simulink model "wind1000_BUS_Original"  (R2024a) (continued)
== system wind1000_BUS_Original/Subsystem_10/String1/WT6 ==
  V_in : Inport
  I_in : Inport  Port=2
  Add1 : Sum  Inputs=+-
  C_cable : Subsystem
  C_filter : Subsystem
  Cable line : Subsystem
  Gain : Gain  Gain=1/parGrid.WTstring.Rparallel_cable
  Transformer-complex : Subsystem
  WFG : Subsystem
  V_out : Outport
  I_out : Outport  Port=2
  wires:
  I_in/1 -> C_cable/2(I_in)
  Gain/1 -> Add1/2
  Add1/1 -> C_cable/1(It2)
  Transformer-complex/2(It2) -> Add1/1
  V_in/1 -> Cable line/1(V)
  Transformer-complex/1(It1) -> C_filter/2(It1)
  Cable line/1(I_cable) -> C_cable/3(I_cable), I_out/1
  C_filter/1(Vc_filter_qd) -> WFG/1(vcqd), Transformer-complex/1(Vc_filter_qd)
  WFG/1(ilqd) -> C_filter/1(IWF)
  C_cable/1(Vc_cable) -> Cable line/2(Vc_cable), V_out/1, Transformer-complex/2(Vc_cable_qd), Gain/1

== system wind1000_BUS_Original/Subsystem_10/String1/WT7 ==
  V_in : Inport
  I_in : Inport  Port=2
  Add1 : Sum  Inputs=+-
  C_cable : Subsystem
  C_filter : Subsystem
  Cable line : Subsystem
  Gain : Gain  Gain=1/parGrid.WTstring.Rparallel_cable
  Transformer-complex : Subsystem
  WFG : Subsystem
  V_out : Outport
  I_out : Outport  Port=2
  wires:
  C_cable/1(Vc_cable) -> Gain/1, Transformer-complex/2(Vc_cable_qd), V_out/1, Cable line/2(Vc_cable)
  WFG/1(ilqd) -> C_filter/1(IWF)
  C_filter/1(Vc_filter_qd) -> WFG/1(vcqd), Transformer-complex/1(Vc_filter_qd)
  Cable line/1(I_cable) -> I_out/1, C_cable/3(I_cable)
  Transformer-complex/1(It1) -> C_filter/2(It1)
  V_in/1 -> Cable line/1(V)
  Transformer-complex/2(It2) -> Add1/1
  Add1/1 -> C_cable/1(It2)
  Gain/1 -> Add1/2
  I_in/1 -> C_cable/2(I_in)

== system wind1000_BUS_Original/Subsystem_10/String1/WT8 ==
  V_in : Inport
  I_in : Inport  Port=2
  Add1 : Sum  Inputs=+-
  C_cable : Subsystem
  C_filter : Subsystem
  Cable line : Subsystem
  Gain : Gain  Gain=1/parGrid.WTstring.Rparallel_cable
  Transformer-complex : Subsystem
  WFG : Subsystem
  V_out : Outport
  I_out : Outport  Port=2
  wires:
  I_in/1 -> C_cable/2(I_in)
  Gain/1 -> Add1/2
  Add1/1 -> C_cable/1(It2)
  Transformer-complex/2(It2) -> Add1/1
  V_in/1 -> Cable line/1(V)
  Transformer-complex/1(It1) -> C_filter/2(It1)
  Cable line/1(I_cable) -> C_cable/3(I_cable), I_out/1
  C_filter/1(Vc_filter_qd) -> WFG/1(vcqd), Transformer-complex/1(Vc_filter_qd)
  WFG/1(ilqd) -> C_filter/1(IWF)
  C_cable/1(Vc_cable) -> Cable line/2(Vc_cable), V_out/1, Transformer-complex/2(Vc_cable_qd), Gain/1

== system wind1000_BUS_Original/Subsystem_10/String1/WT9 ==
  V_in : Inport
  I_in : Inport  Port=2
  Add1 : Sum  Inputs=+-
  C_cable : Subsystem
  C_filter : Subsystem
  Cable line : Subsystem
  Gain : Gain  Gain=1/parGrid.WTstring.Rparallel_cable
  Transformer-complex : Subsystem
  WFG : Subsystem
  V_out : Outport
  I_out : Outport  Port=2
  wires:
  C_cable/1(Vc_cable) -> Gain/1, Transformer-complex/2(Vc_cable_qd), V_out/1, Cable line/2(Vc_cable)
  WFG/1(ilqd) -> C_filter/1(IWF)
  C_filter/1(Vc_filter_qd) -> WFG/1(vcqd), Transformer-complex/1(Vc_filter_qd)
  Cable line/1(I_cable) -> I_out/1, C_cable/3(I_cable)
  Transformer-complex/1(It1) -> C_filter/2(It1)
  V_in/1 -> Cable line/1(V)
  Transformer-complex/2(It2) -> Add1/1
  Add1/1 -> C_cable/1(It2)
  Gain/1 -> Add1/2
  I_in/1 -> C_cable/2(I_in)

== system wind1000_BUS_Original/Subsystem_20/3phase2dq/Font trifasica asimetrica1 ==
  t : Inport
  Clock : Clock
  Constant1 : Constant  Value=phi_D
  Constant2 : Constant  Value=phi_I
  Constant3 : Constant  Value=2*pi/3
  Constant4 : Constant  Value=2*pi/3
  Gain : Gain  Gain=we
  Gain1 : Gain  Gain=A_D
  Gain2 : Gain  Gain=A_I
  Mux : Mux  Inputs=3
  Mux1 : Mux  Inputs=3
  Park : Subsystem
  Product : Product  RndMeth=Zero
  Product1 : Product  RndMeth=Zero
  Product4 : Product  Multiplication=Matrix(*)  RndMeth=Zero
  Scope1 : Scope
  Sum{1,3,5..7} (5 alike) : Sum  Inputs=|++
  Sum2 : Sum  Inputs=|+-
  Sum4 : Sum  Inputs=|+-
  Trigonometric Function{1..6} (6 alike) : Trigonometry  Operator=cos
  directa : Lookup  InputValues=tt_D  Table=tA_D  SaturateOnIntegerOverflow=off
  inversa : Lookup  InputValues=tt_I  Table=tA_I  SaturateOnIntegerOverflow=off
  we : Gain  Gain=100*pi
  y : Outport
  wires:
  Sum3/1 -> Trigonometric Function2/1, Sum2/1, Sum1/1
  Sum2/1 -> Trigonometric Function1/1
  Sum1/1 -> Trigonometric Function3/1
  Constant4/1 -> Sum2/2, Sum1/2
  Trigonometric Function2/1 -> Mux/1
  Trigonometric Function1/1 -> Mux/2
  Trigonometric Function3/1 -> Mux/3
  Mux/1 -> Gain1/1
  t/1 -> Gain/1, directa/1, inversa/1
  Gain/1 -> Sum3/1, Sum6/1
  Constant1/1 -> Sum3/2
  Sum6/1 -> Trigonometric Function5/1, Sum5/1, Sum4/1
  Sum5/1 -> Trigonometric Function4/1
  Sum4/1 -> Trigonometric Function6/1
  Constant3/1 -> Sum4/2, Sum5/2
  Trigonometric Function5/1 -> Mux1/1
  Trigonometric Function4/1 -> Mux1/2
  Trigonometric Function6/1 -> Mux1/3
  Mux1/1 -> Gain2/1
  Constant2/1 -> Sum6/2
  Product/1 -> Sum7/1
  directa/1 -> Product/1
  inversa/1 -> Product1/1
  Gain1/1 -> Product/2
  Gain2/1 -> Product1/2
  Product1/1 -> Sum7/2
  Park/1(Tthetar) -> Scope1/1, Product4/1
  Clock/1 -> we/1
  we/1 -> Park/1(theta_r)
  Sum7/1 -> Product4/2
  Product4/1 -> y/1

== system wind1000_BUS_Original/Subsystem_20/Subsystem/String1 ==
  V_in : Inport
  Constant : Constant  Value=0
  Scope : Scope
  WT1 : Subsystem
  WT10 : Subsystem
  WT2 : Subsystem
  WT3 : Subsystem
  WT4 : Subsystem
  WT5 : Subsystem
  WT6 : Subsystem
  WT7 : Subsystem
  WT8 : Subsystem
  WT9 : Subsystem
  I_out : Outport
  wires:
  WT10/1(V_out) -> Scope/1
  WT7/1(V_out) -> WT8/1(V_in)
  Constant/1 -> WT10/2(I_in)
  WT4/1(V_out) -> WT5/1(V_in)
  WT4/2(I_out) -> WT3/2(I_in)
  WT3/1(V_out) -> WT4/1(V_in)
  WT1/1(V_out) -> WT2/1(V_in)
  WT10/2(I_out) -> WT9/2(I_in)
  WT5/1(V_out) -> WT6/1(V_in)
  WT2/1(V_out) -> WT3/1(V_in)
  WT7/2(I_out) -> WT6/2(I_in)
  WT8/1(V_out) -> WT9/1(V_in)
  WT6/1(V_out) -> WT7/1(V_in)
  WT9/2(I_out) -> WT8/2(I_in)
  WT5/2(I_out) -> WT4/2(I_in)
  WT2/2(I_out) -> WT1/2(I_in)
  V_in/1 -> WT1/1(V_in)
  WT3/2(I_out) -> WT2/2(I_in)
  WT8/2(I_out) -> WT7/2(I_in)
  WT9/1(V_out) -> WT10/1(V_in)
  WT6/2(I_out) -> WT5/2(I_in)
  WT1/2(I_out) -> I_out/1

== system wind1000_BUS_Original/Subsystem_20/Subsystem/String2 ==
  V_in : Inport
  Constant : Constant  Value=0
  Scope : Scope
  WT1 : Subsystem
  WT10 : Subsystem
  WT2 : Subsystem
  WT3 : Subsystem
  WT4 : Subsystem
  WT5 : Subsystem
  WT6 : Subsystem
  WT7 : Subsystem
  WT8 : Subsystem
  WT9 : Subsystem
  I_out : Outport
  wires:
  WT1/2(I_out) -> I_out/1
  WT6/2(I_out) -> WT5/2(I_in)
  WT9/1(V_out) -> WT10/1(V_in)
  WT8/2(I_out) -> WT7/2(I_in)
  WT3/2(I_out) -> WT2/2(I_in)
  V_in/1 -> WT1/1(V_in)
  WT2/2(I_out) -> WT1/2(I_in)
  WT5/2(I_out) -> WT4/2(I_in)
  WT9/2(I_out) -> WT8/2(I_in)
  WT6/1(V_out) -> WT7/1(V_in)
  WT8/1(V_out) -> WT9/1(V_in)
  WT7/2(I_out) -> WT6/2(I_in)
  WT2/1(V_out) -> WT3/1(V_in)
  WT5/1(V_out) -> WT6/1(V_in)
  WT10/2(I_out) -> WT9/2(I_in)
  WT1/1(V_out) -> WT2/1(V_in)
  WT3/1(V_out) -> WT4/1(V_in)
  WT4/2(I_out) -> WT3/2(I_in)
  WT4/1(V_out) -> WT5/1(V_in)
  Constant/1 -> WT10/2(I_in)
  WT7/1(V_out) -> WT8/1(V_in)
  WT10/1(V_out) -> Scope/1

== system wind1000_BUS_Original/Subsystem_20/Subsystem/String3 ==
  V_in : Inport
  Constant : Constant  Value=0
  Scope : Scope
  WT1 : Subsystem
  WT10 : Subsystem
  WT2 : Subsystem
  WT3 : Subsystem
  WT4 : Subsystem
  WT5 : Subsystem
  WT6 : Subsystem
  WT7 : Subsystem
  WT8 : Subsystem
  WT9 : Subsystem
  I_out : Outport
  wires:
  WT1/2(I_out) -> I_out/1
  WT6/2(I_out) -> WT5/2(I_in)
  WT9/1(V_out) -> WT10/1(V_in)
  WT8/2(I_out) -> WT7/2(I_in)
  WT3/2(I_out) -> WT2/2(I_in)
  V_in/1 -> WT1/1(V_in)
  WT2/2(I_out) -> WT1/2(I_in)
  WT5/2(I_out) -> WT4/2(I_in)
  WT9/2(I_out) -> WT8/2(I_in)
  WT6/1(V_out) -> WT7/1(V_in)
  WT8/1(V_out) -> WT9/1(V_in)
  WT7/2(I_out) -> WT6/2(I_in)
  WT2/1(V_out) -> WT3/1(V_in)
  WT5/1(V_out) -> WT6/1(V_in)
  WT10/2(I_out) -> WT9/2(I_in)
  WT1/1(V_out) -> WT2/1(V_in)
  WT3/1(V_out) -> WT4/1(V_in)
  WT4/2(I_out) -> WT3/2(I_in)
  WT4/1(V_out) -> WT5/1(V_in)
  Constant/1 -> WT10/2(I_in)
  WT7/1(V_out) -> WT8/1(V_in)
  WT10/1(V_out) -> Scope/1

== system wind1000_BUS_Original/Subsystem_20/Subsystem/String4 ==
  V_in : Inport
  Constant : Constant  Value=0
  Scope : Scope
  WT1 : Subsystem
  WT10 : Subsystem
  WT2 : Subsystem
  WT3 : Subsystem
  WT4 : Subsystem
  WT5 : Subsystem
  WT6 : Subsystem
  WT7 : Subsystem
  WT8 : Subsystem
  WT9 : Subsystem
  I_out : Outport
  wires:
  WT1/2(I_out) -> I_out/1
  WT6/2(I_out) -> WT5/2(I_in)
  WT9/1(V_out) -> WT10/1(V_in)
  WT8/2(I_out) -> WT7/2(I_in)
  WT3/2(I_out) -> WT2/2(I_in)
  V_in/1 -> WT1/1(V_in)
  WT2/2(I_out) -> WT1/2(I_in)
  WT5/2(I_out) -> WT4/2(I_in)
  WT9/2(I_out) -> WT8/2(I_in)
  WT6/1(V_out) -> WT7/1(V_in)
  WT8/1(V_out) -> WT9/1(V_in)
  WT7/2(I_out) -> WT6/2(I_in)
  WT2/1(V_out) -> WT3/1(V_in)
  WT5/1(V_out) -> WT6/1(V_in)
  WT10/2(I_out) -> WT9/2(I_in)
  WT1/1(V_out) -> WT2/1(V_in)
  WT3/1(V_out) -> WT4/1(V_in)
  WT4/2(I_out) -> WT3/2(I_in)
  WT4/1(V_out) -> WT5/1(V_in)
  Constant/1 -> WT10/2(I_in)
  WT7/1(V_out) -> WT8/1(V_in)
  WT10/1(V_out) -> Scope/1

== system wind1000_BUS_Original/Subsystem_20/Subsystem/String5 ==
  V_in : Inport
  Constant : Constant  Value=0
  Scope : Scope
  WT1 : Subsystem
  WT10 : Subsystem
  WT2 : Subsystem
  WT3 : Subsystem
  WT4 : Subsystem
  WT5 : Subsystem
  WT6 : Subsystem
  WT7 : Subsystem
  WT8 : Subsystem
  WT9 : Subsystem
  I_out : Outport
  wires:
  WT1/2(I_out) -> I_out/1
  WT6/2(I_out) -> WT5/2(I_in)
  WT9/1(V_out) -> WT10/1(V_in)
  WT8/2(I_out) -> WT7/2(I_in)
  WT3/2(I_out) -> WT2/2(I_in)
  V_in/1 -> WT1/1(V_in)
  WT2/2(I_out) -> WT1/2(I_in)
  WT5/2(I_out) -> WT4/2(I_in)
  WT9/2(I_out) -> WT8/2(I_in)
  WT6/1(V_out) -> WT7/1(V_in)
  WT8/1(V_out) -> WT9/1(V_in)
  WT7/2(I_out) -> WT6/2(I_in)
  WT2/1(V_out) -> WT3/1(V_in)
  WT5/1(V_out) -> WT6/1(V_in)
  WT10/2(I_out) -> WT9/2(I_in)
  WT1/1(V_out) -> WT2/1(V_in)
  WT3/1(V_out) -> WT4/1(V_in)
  WT4/2(I_out) -> WT3/2(I_in)
  WT4/1(V_out) -> WT5/1(V_in)
  Constant/1 -> WT10/2(I_in)
  WT7/1(V_out) -> WT8/1(V_in)
  WT10/1(V_out) -> Scope/1

== system wind1000_BUS_Original/Subsystem_20/Subsystem/String6 ==
  V_in : Inport
  Constant : Constant  Value=0
  Scope : Scope
  WT1 : Subsystem
  WT10 : Subsystem
  WT2 : Subsystem
  WT3 : Subsystem
  WT4 : Subsystem
  WT5 : Subsystem
  WT6 : Subsystem
  WT7 : Subsystem
  WT8 : Subsystem
  WT9 : Subsystem
  I_out : Outport
  wires:
  WT1/2(I_out) -> I_out/1
  WT6/2(I_out) -> WT5/2(I_in)
  WT9/1(V_out) -> WT10/1(V_in)
  WT8/2(I_out) -> WT7/2(I_in)
  WT3/2(I_out) -> WT2/2(I_in)
  V_in/1 -> WT1/1(V_in)
  WT2/2(I_out) -> WT1/2(I_in)
  WT5/2(I_out) -> WT4/2(I_in)
  WT9/2(I_out) -> WT8/2(I_in)
  WT6/1(V_out) -> WT7/1(V_in)
  WT8/1(V_out) -> WT9/1(V_in)
  WT7/2(I_out) -> WT6/2(I_in)
  WT2/1(V_out) -> WT3/1(V_in)
  WT5/1(V_out) -> WT6/1(V_in)
  WT10/2(I_out) -> WT9/2(I_in)
  WT1/1(V_out) -> WT2/1(V_in)
  WT3/1(V_out) -> WT4/1(V_in)
  WT4/2(I_out) -> WT3/2(I_in)
  WT4/1(V_out) -> WT5/1(V_in)
  Constant/1 -> WT10/2(I_in)
  WT7/1(V_out) -> WT8/1(V_in)
  WT10/1(V_out) -> Scope/1

== system wind1000_BUS_Original/Subsystem_200/3phase2dq/Font trifasica asimetrica1 ==
  t : Inport
  Clock : Clock
  Constant1 : Constant  Value=phi_D
  Constant2 : Constant  Value=phi_I
  Constant3 : Constant  Value=2*pi/3
  Constant4 : Constant  Value=2*pi/3
  Gain : Gain  Gain=we
  Gain1 : Gain  Gain=A_D
  Gain2 : Gain  Gain=A_I
  Mux : Mux  Inputs=3
  Mux1 : Mux  Inputs=3
  Park : Subsystem
  Product : Product  RndMeth=Zero
  Product1 : Product  RndMeth=Zero
  Product4 : Product  Multiplication=Matrix(*)  RndMeth=Zero
  Scope1 : Scope
  Sum{1,3,5..7} (5 alike) : Sum  Inputs=|++
  Sum2 : Sum  Inputs=|+-
  Sum4 : Sum  Inputs=|+-
  Trigonometric Function{1..6} (6 alike) : Trigonometry  Operator=cos
  directa : Lookup  InputValues=tt_D  Table=tA_D  SaturateOnIntegerOverflow=off
  inversa : Lookup  InputValues=tt_I  Table=tA_I  SaturateOnIntegerOverflow=off
  we : Gain  Gain=100*pi
  y : Outport
  wires:
  Sum3/1 -> Trigonometric Function2/1, Sum2/1, Sum1/1
  Sum2/1 -> Trigonometric Function1/1
  Sum1/1 -> Trigonometric Function3/1
  Constant4/1 -> Sum2/2, Sum1/2
  Trigonometric Function2/1 -> Mux/1
  Trigonometric Function1/1 -> Mux/2
  Trigonometric Function3/1 -> Mux/3
  Mux/1 -> Gain1/1
  t/1 -> Gain/1, directa/1, inversa/1
  Gain/1 -> Sum3/1, Sum6/1
  Constant1/1 -> Sum3/2
  Sum6/1 -> Trigonometric Function5/1, Sum5/1, Sum4/1
  Sum5/1 -> Trigonometric Function4/1
  Sum4/1 -> Trigonometric Function6/1
  Constant3/1 -> Sum4/2, Sum5/2
  Trigonometric Function5/1 -> Mux1/1
  Trigonometric Function4/1 -> Mux1/2
  Trigonometric Function6/1 -> Mux1/3
  Mux1/1 -> Gain2/1
  Constant2/1 -> Sum6/2
  Product/1 -> Sum7/1
  directa/1 -> Product/1
  inversa/1 -> Product1/1
  Gain1/1 -> Product/2
  Gain2/1 -> Product1/2
  Product1/1 -> Sum7/2
  Park/1(Tthetar) -> Scope1/1, Product4/1
  Clock/1 -> we/1
  we/1 -> Park/1(theta_r)
  Sum7/1 -> Product4/2
  Product4/1 -> y/1

== system wind1000_BUS_Original/Subsystem_200/Subsystem/String1 ==
  V_in : Inport
  Constant : Constant  Value=0
  Scope : Scope
  WT1 : Subsystem
  WT10 : Subsystem
  WT2 : Subsystem
  WT3 : Subsystem
  WT4 : Subsystem
  WT5 : Subsystem
  WT6 : Subsystem
  WT7 : Subsystem
  WT8 : Subsystem
  WT9 : Subsystem
  I_out : Outport
  wires:
  WT10/1(V_out) -> Scope/1
  WT7/1(V_out) -> WT8/1(V_in)
  Constant/1 -> WT10/2(I_in)
  WT4/1(V_out) -> WT5/1(V_in)
  WT4/2(I_out) -> WT3/2(I_in)
  WT3/1(V_out) -> WT4/1(V_in)
  WT1/1(V_out) -> WT2/1(V_in)
  WT10/2(I_out) -> WT9/2(I_in)
  WT5/1(V_out) -> WT6/1(V_in)
  WT2/1(V_out) -> WT3/1(V_in)
  WT7/2(I_out) -> WT6/2(I_in)
  WT8/1(V_out) -> WT9/1(V_in)
  WT6/1(V_out) -> WT7/1(V_in)
  WT9/2(I_out) -> WT8/2(I_in)
  WT5/2(I_out) -> WT4/2(I_in)
  WT2/2(I_out) -> WT1/2(I_in)
  V_in/1 -> WT1/1(V_in)
  WT3/2(I_out) -> WT2/2(I_in)
  WT8/2(I_out) -> WT7/2(I_in)
  WT9/1(V_out) -> WT10/1(V_in)
  WT6/2(I_out) -> WT5/2(I_in)
  WT1/2(I_out) -> I_out/1

== system wind1000_BUS_Original/Subsystem_200/Subsystem/String10 ==
  V_in : Inport
  Constant : Constant  Value=0
  Scope : Scope
  WT1 : Subsystem
  WT10 : Subsystem
  WT2 : Subsystem
  WT3 : Subsystem
  WT4 : Subsystem
  WT5 : Subsystem
  WT6 : Subsystem
  WT7 : Subsystem
  WT8 : Subsystem
  WT9 : Subsystem
  I_out : Outport
  wires:
  WT1/2(I_out) -> I_out/1
  WT6/2(I_out) -> WT5/2(I_in)
  WT9/1(V_out) -> WT10/1(V_in)
  WT8/2(I_out) -> WT7/2(I_in)
  WT3/2(I_out) -> WT2/2(I_in)
  V_in/1 -> WT1/1(V_in)
  WT2/2(I_out) -> WT1/2(I_in)
  WT5/2(I_out) -> WT4/2(I_in)
  WT9/2(I_out) -> WT8/2(I_in)
  WT6/1(V_out) -> WT7/1(V_in)
  WT8/1(V_out) -> WT9/1(V_in)
  WT7/2(I_out) -> WT6/2(I_in)
  WT2/1(V_out) -> WT3/1(V_in)
  WT5/1(V_out) -> WT6/1(V_in)
  WT10/2(I_out) -> WT9/2(I_in)
  WT1/1(V_out) -> WT2/1(V_in)
  WT3/1(V_out) -> WT4/1(V_in)
  WT4/2(I_out) -> WT3/2(I_in)
  WT4/1(V_out) -> WT5/1(V_in)
  Constant/1 -> WT10/2(I_in)
  WT7/1(V_out) -> WT8/1(V_in)
  WT10/1(V_out) -> Scope/1

== system wind1000_BUS_Original/Subsystem_200/Subsystem/String11 ==
  V_in : Inport
  Constant : Constant  Value=0
  Scope : Scope
  WT1 : Subsystem
  WT10 : Subsystem
  WT2 : Subsystem
  WT3 : Subsystem
  WT4 : Subsystem
  WT5 : Subsystem
  WT6 : Subsystem
  WT7 : Subsystem
  WT8 : Subsystem
  WT9 : Subsystem
  I_out : Outport
  wires:
  WT1/2(I_out) -> I_out/1
  WT6/2(I_out) -> WT5/2(I_in)
  WT9/1(V_out) -> WT10/1(V_in)
  WT8/2(I_out) -> WT7/2(I_in)
  WT3/2(I_out) -> WT2/2(I_in)
  V_in/1 -> WT1/1(V_in)
  WT2/2(I_out) -> WT1/2(I_in)
  WT5/2(I_out) -> WT4/2(I_in)
  WT9/2(I_out) -> WT8/2(I_in)
  WT6/1(V_out) -> WT7/1(V_in)
  WT8/1(V_out) -> WT9/1(V_in)
  WT7/2(I_out) -> WT6/2(I_in)
  WT2/1(V_out) -> WT3/1(V_in)
  WT5/1(V_out) -> WT6/1(V_in)
  WT10/2(I_out) -> WT9/2(I_in)
  WT1/1(V_out) -> WT2/1(V_in)
  WT3/1(V_out) -> WT4/1(V_in)
  WT4/2(I_out) -> WT3/2(I_in)
  WT4/1(V_out) -> WT5/1(V_in)
  Constant/1 -> WT10/2(I_in)
  WT7/1(V_out) -> WT8/1(V_in)
  WT10/1(V_out) -> Scope/1

== system wind1000_BUS_Original/Subsystem_200/Subsystem/String12 ==
  V_in : Inport
  Constant : Constant  Value=0
  Scope : Scope
  WT1 : Subsystem
  WT10 : Subsystem
  WT2 : Subsystem
  WT3 : Subsystem
  WT4 : Subsystem
  WT5 : Subsystem
  WT6 : Subsystem
  WT7 : Subsystem
  WT8 : Subsystem
  WT9 : Subsystem
  I_out : Outport
  wires:
  WT1/2(I_out) -> I_out/1
  WT6/2(I_out) -> WT5/2(I_in)
  WT9/1(V_out) -> WT10/1(V_in)
  WT8/2(I_out) -> WT7/2(I_in)
  WT3/2(I_out) -> WT2/2(I_in)
  V_in/1 -> WT1/1(V_in)
  WT2/2(I_out) -> WT1/2(I_in)
  WT5/2(I_out) -> WT4/2(I_in)
  WT9/2(I_out) -> WT8/2(I_in)
  WT6/1(V_out) -> WT7/1(V_in)
  WT8/1(V_out) -> WT9/1(V_in)
  WT7/2(I_out) -> WT6/2(I_in)
  WT2/1(V_out) -> WT3/1(V_in)
  WT5/1(V_out) -> WT6/1(V_in)
  WT10/2(I_out) -> WT9/2(I_in)
  WT1/1(V_out) -> WT2/1(V_in)
  WT3/1(V_out) -> WT4/1(V_in)
  WT4/2(I_out) -> WT3/2(I_in)
  WT4/1(V_out) -> WT5/1(V_in)
  Constant/1 -> WT10/2(I_in)
  WT7/1(V_out) -> WT8/1(V_in)
  WT10/1(V_out) -> Scope/1

== system wind1000_BUS_Original/Subsystem_200/Subsystem/String13 ==
  V_in : Inport
  Constant : Constant  Value=0
  Scope : Scope
  WT1 : Subsystem
  WT10 : Subsystem
  WT2 : Subsystem
  WT3 : Subsystem
  WT4 : Subsystem
  WT5 : Subsystem
  WT6 : Subsystem
  WT7 : Subsystem
  WT8 : Subsystem
  WT9 : Subsystem
  I_out : Outport
  wires:
  WT1/2(I_out) -> I_out/1
  WT6/2(I_out) -> WT5/2(I_in)
  WT9/1(V_out) -> WT10/1(V_in)
  WT8/2(I_out) -> WT7/2(I_in)
  WT3/2(I_out) -> WT2/2(I_in)
  V_in/1 -> WT1/1(V_in)
  WT2/2(I_out) -> WT1/2(I_in)
  WT5/2(I_out) -> WT4/2(I_in)
  WT9/2(I_out) -> WT8/2(I_in)
  WT6/1(V_out) -> WT7/1(V_in)
  WT8/1(V_out) -> WT9/1(V_in)
  WT7/2(I_out) -> WT6/2(I_in)
  WT2/1(V_out) -> WT3/1(V_in)
  WT5/1(V_out) -> WT6/1(V_in)
  WT10/2(I_out) -> WT9/2(I_in)
  WT1/1(V_out) -> WT2/1(V_in)
  WT3/1(V_out) -> WT4/1(V_in)
  WT4/2(I_out) -> WT3/2(I_in)
  WT4/1(V_out) -> WT5/1(V_in)
  Constant/1 -> WT10/2(I_in)
  WT7/1(V_out) -> WT8/1(V_in)
  WT10/1(V_out) -> Scope/1

== system wind1000_BUS_Original/Subsystem_200/Subsystem/String14 ==
  V_in : Inport
  Constant : Constant  Value=0
  Scope : Scope
  WT1 : Subsystem
  WT10 : Subsystem
  WT2 : Subsystem
  WT3 : Subsystem
  WT4 : Subsystem
  WT5 : Subsystem
  WT6 : Subsystem
  WT7 : Subsystem
  WT8 : Subsystem
  WT9 : Subsystem
  I_out : Outport
  wires:
  WT1/2(I_out) -> I_out/1
  WT6/2(I_out) -> WT5/2(I_in)
  WT9/1(V_out) -> WT10/1(V_in)
  WT8/2(I_out) -> WT7/2(I_in)
  WT3/2(I_out) -> WT2/2(I_in)
  V_in/1 -> WT1/1(V_in)
  WT2/2(I_out) -> WT1/2(I_in)
  WT5/2(I_out) -> WT4/2(I_in)
  WT9/2(I_out) -> WT8/2(I_in)
  WT6/1(V_out) -> WT7/1(V_in)
  WT8/1(V_out) -> WT9/1(V_in)
  WT7/2(I_out) -> WT6/2(I_in)
  WT2/1(V_out) -> WT3/1(V_in)
  WT5/1(V_out) -> WT6/1(V_in)
  WT10/2(I_out) -> WT9/2(I_in)
  WT1/1(V_out) -> WT2/1(V_in)
  WT3/1(V_out) -> WT4/1(V_in)
  WT4/2(I_out) -> WT3/2(I_in)
  WT4/1(V_out) -> WT5/1(V_in)
  Constant/1 -> WT10/2(I_in)
  WT7/1(V_out) -> WT8/1(V_in)
  WT10/1(V_out) -> Scope/1

== system wind1000_BUS_Original/Subsystem_200/Subsystem/String15 ==
  V_in : Inport
  Constant : Constant  Value=0
  Scope : Scope
  WT1 : Subsystem
  WT10 : Subsystem
  WT2 : Subsystem
  WT3 : Subsystem
  WT4 : Subsystem
  WT5 : Subsystem
  WT6 : Subsystem
  WT7 : Subsystem
  WT8 : Subsystem
  WT9 : Subsystem
  I_out : Outport
  wires:
  WT1/2(I_out) -> I_out/1
  WT6/2(I_out) -> WT5/2(I_in)
  WT9/1(V_out) -> WT10/1(V_in)
  WT8/2(I_out) -> WT7/2(I_in)
  WT3/2(I_out) -> WT2/2(I_in)
  V_in/1 -> WT1/1(V_in)
  WT2/2(I_out) -> WT1/2(I_in)
  WT5/2(I_out) -> WT4/2(I_in)
  WT9/2(I_out) -> WT8/2(I_in)
  WT6/1(V_out) -> WT7/1(V_in)
  WT8/1(V_out) -> WT9/1(V_in)
  WT7/2(I_out) -> WT6/2(I_in)
  WT2/1(V_out) -> WT3/1(V_in)
  WT5/1(V_out) -> WT6/1(V_in)
  WT10/2(I_out) -> WT9/2(I_in)
  WT1/1(V_out) -> WT2/1(V_in)
  WT3/1(V_out) -> WT4/1(V_in)
  WT4/2(I_out) -> WT3/2(I_in)
  WT4/1(V_out) -> WT5/1(V_in)
  Constant/1 -> WT10/2(I_in)
  WT7/1(V_out) -> WT8/1(V_in)
  WT10/1(V_out) -> Scope/1

== system wind1000_BUS_Original/Subsystem_200/Subsystem/String16 ==
  V_in : Inport
  Constant : Constant  Value=0
  Scope : Scope
  WT1 : Subsystem
  WT10 : Subsystem
  WT2 : Subsystem
  WT3 : Subsystem
  WT4 : Subsystem
  WT5 : Subsystem
  WT6 : Subsystem
  WT7 : Subsystem
  WT8 : Subsystem
  WT9 : Subsystem
  I_out : Outport
  wires:
  WT1/2(I_out) -> I_out/1
  WT6/2(I_out) -> WT5/2(I_in)
  WT9/1(V_out) -> WT10/1(V_in)
  WT8/2(I_out) -> WT7/2(I_in)
  WT3/2(I_out) -> WT2/2(I_in)
  V_in/1 -> WT1/1(V_in)
  WT2/2(I_out) -> WT1/2(I_in)
  WT5/2(I_out) -> WT4/2(I_in)
  WT9/2(I_out) -> WT8/2(I_in)
  WT6/1(V_out) -> WT7/1(V_in)
  WT8/1(V_out) -> WT9/1(V_in)
  WT7/2(I_out) -> WT6/2(I_in)
  WT2/1(V_out) -> WT3/1(V_in)
  WT5/1(V_out) -> WT6/1(V_in)
  WT10/2(I_out) -> WT9/2(I_in)
  WT1/1(V_out) -> WT2/1(V_in)
  WT3/1(V_out) -> WT4/1(V_in)
  WT4/2(I_out) -> WT3/2(I_in)
  WT4/1(V_out) -> WT5/1(V_in)
  Constant/1 -> WT10/2(I_in)
  WT7/1(V_out) -> WT8/1(V_in)
  WT10/1(V_out) -> Scope/1

== system wind1000_BUS_Original/Subsystem_200/Subsystem/String17 ==
  V_in : Inport
  Constant : Constant  Value=0
  Scope : Scope
  WT1 : Subsystem
  WT10 : Subsystem
  WT2 : Subsystem
  WT3 : Subsystem
  WT4 : Subsystem
  WT5 : Subsystem
  WT6 : Subsystem
  WT7 : Subsystem
  WT8 : Subsystem
  WT9 : Subsystem
  I_out : Outport
  wires:
  WT1/2(I_out) -> I_out/1
  WT6/2(I_out) -> WT5/2(I_in)
  WT9/1(V_out) -> WT10/1(V_in)
  WT8/2(I_out) -> WT7/2(I_in)
  WT3/2(I_out) -> WT2/2(I_in)
  V_in/1 -> WT1/1(V_in)
  WT2/2(I_out) -> WT1/2(I_in)
  WT5/2(I_out) -> WT4/2(I_in)
  WT9/2(I_out) -> WT8/2(I_in)
  WT6/1(V_out) -> WT7/1(V_in)
  WT8/1(V_out) -> WT9/1(V_in)
  WT7/2(I_out) -> WT6/2(I_in)
  WT2/1(V_out) -> WT3/1(V_in)
  WT5/1(V_out) -> WT6/1(V_in)
  WT10/2(I_out) -> WT9/2(I_in)
  WT1/1(V_out) -> WT2/1(V_in)
  WT3/1(V_out) -> WT4/1(V_in)
  WT4/2(I_out) -> WT3/2(I_in)
  WT4/1(V_out) -> WT5/1(V_in)
  Constant/1 -> WT10/2(I_in)
  WT7/1(V_out) -> WT8/1(V_in)
  WT10/1(V_out) -> Scope/1

== system wind1000_BUS_Original/Subsystem_200/Subsystem/String18 ==
  V_in : Inport
  Constant : Constant  Value=0
  Scope : Scope
  WT1 : Subsystem
  WT10 : Subsystem
  WT2 : Subsystem
  WT3 : Subsystem
  WT4 : Subsystem
  WT5 : Subsystem
  WT6 : Subsystem
  WT7 : Subsystem
  WT8 : Subsystem
  WT9 : Subsystem
  I_out : Outport
  wires:
  WT1/2(I_out) -> I_out/1
  WT6/2(I_out) -> WT5/2(I_in)
  WT9/1(V_out) -> WT10/1(V_in)
  WT8/2(I_out) -> WT7/2(I_in)
  WT3/2(I_out) -> WT2/2(I_in)
  V_in/1 -> WT1/1(V_in)
  WT2/2(I_out) -> WT1/2(I_in)
  WT5/2(I_out) -> WT4/2(I_in)
  WT9/2(I_out) -> WT8/2(I_in)
  WT6/1(V_out) -> WT7/1(V_in)
  WT8/1(V_out) -> WT9/1(V_in)
  WT7/2(I_out) -> WT6/2(I_in)
  WT2/1(V_out) -> WT3/1(V_in)
  WT5/1(V_out) -> WT6/1(V_in)
  WT10/2(I_out) -> WT9/2(I_in)
  WT1/1(V_out) -> WT2/1(V_in)
  WT3/1(V_out) -> WT4/1(V_in)
  WT4/2(I_out) -> WT3/2(I_in)
  WT4/1(V_out) -> WT5/1(V_in)
  Constant/1 -> WT10/2(I_in)
  WT7/1(V_out) -> WT8/1(V_in)
  WT10/1(V_out) -> Scope/1

== system wind1000_BUS_Original/Subsystem_200/Subsystem/String19 ==
  V_in : Inport
  Constant : Constant  Value=0
  Scope : Scope
  WT1 : Subsystem
  WT10 : Subsystem
  WT2 : Subsystem
  WT3 : Subsystem
  WT4 : Subsystem
  WT5 : Subsystem
  WT6 : Subsystem
  WT7 : Subsystem
  WT8 : Subsystem
  WT9 : Subsystem
  I_out : Outport
  wires:
  WT1/2(I_out) -> I_out/1
  WT6/2(I_out) -> WT5/2(I_in)
  WT9/1(V_out) -> WT10/1(V_in)
  WT8/2(I_out) -> WT7/2(I_in)
  WT3/2(I_out) -> WT2/2(I_in)
  V_in/1 -> WT1/1(V_in)
  WT2/2(I_out) -> WT1/2(I_in)
  WT5/2(I_out) -> WT4/2(I_in)
  WT9/2(I_out) -> WT8/2(I_in)
  WT6/1(V_out) -> WT7/1(V_in)
  WT8/1(V_out) -> WT9/1(V_in)
  WT7/2(I_out) -> WT6/2(I_in)
  WT2/1(V_out) -> WT3/1(V_in)
  WT5/1(V_out) -> WT6/1(V_in)
  WT10/2(I_out) -> WT9/2(I_in)
  WT1/1(V_out) -> WT2/1(V_in)
  WT3/1(V_out) -> WT4/1(V_in)
  WT4/2(I_out) -> WT3/2(I_in)
  WT4/1(V_out) -> WT5/1(V_in)
  Constant/1 -> WT10/2(I_in)
  WT7/1(V_out) -> WT8/1(V_in)
  WT10/1(V_out) -> Scope/1

== system wind1000_BUS_Original/Subsystem_200/Subsystem/String2 ==
  V_in : Inport
  Constant : Constant  Value=0
  Scope : Scope
  WT1 : Subsystem
  WT10 : Subsystem
  WT2 : Subsystem
  WT3 : Subsystem
  WT4 : Subsystem
  WT5 : Subsystem
  WT6 : Subsystem
  WT7 : Subsystem
  WT8 : Subsystem
  WT9 : Subsystem
  I_out : Outport
  wires:
  WT1/2(I_out) -> I_out/1
  WT6/2(I_out) -> WT5/2(I_in)
  WT9/1(V_out) -> WT10/1(V_in)
  WT8/2(I_out) -> WT7/2(I_in)
  WT3/2(I_out) -> WT2/2(I_in)
  V_in/1 -> WT1/1(V_in)
  WT2/2(I_out) -> WT1/2(I_in)
  WT5/2(I_out) -> WT4/2(I_in)
  WT9/2(I_out) -> WT8/2(I_in)
  WT6/1(V_out) -> WT7/1(V_in)
  WT8/1(V_out) -> WT9/1(V_in)
  WT7/2(I_out) -> WT6/2(I_in)
  WT2/1(V_out) -> WT3/1(V_in)
  WT5/1(V_out) -> WT6/1(V_in)
  WT10/2(I_out) -> WT9/2(I_in)
  WT1/1(V_out) -> WT2/1(V_in)
  WT3/1(V_out) -> WT4/1(V_in)
  WT4/2(I_out) -> WT3/2(I_in)
  WT4/1(V_out) -> WT5/1(V_in)
  Constant/1 -> WT10/2(I_in)
  WT7/1(V_out) -> WT8/1(V_in)
  WT10/1(V_out) -> Scope/1

== system wind1000_BUS_Original/Subsystem_200/Subsystem/String20 ==
  V_in : Inport
  Constant : Constant  Value=0
  Scope : Scope
  WT1 : Subsystem
  WT10 : Subsystem
  WT2 : Subsystem
  WT3 : Subsystem
  WT4 : Subsystem
  WT5 : Subsystem
  WT6 : Subsystem
  WT7 : Subsystem
  WT8 : Subsystem
  WT9 : Subsystem
  I_out : Outport
  wires:
  WT1/2(I_out) -> I_out/1
  WT6/2(I_out) -> WT5/2(I_in)
  WT9/1(V_out) -> WT10/1(V_in)
  WT8/2(I_out) -> WT7/2(I_in)
  WT3/2(I_out) -> WT2/2(I_in)
  V_in/1 -> WT1/1(V_in)
  WT2/2(I_out) -> WT1/2(I_in)
  WT5/2(I_out) -> WT4/2(I_in)
  WT9/2(I_out) -> WT8/2(I_in)
  WT6/1(V_out) -> WT7/1(V_in)
  WT8/1(V_out) -> WT9/1(V_in)
  WT7/2(I_out) -> WT6/2(I_in)
  WT2/1(V_out) -> WT3/1(V_in)
  WT5/1(V_out) -> WT6/1(V_in)
  WT10/2(I_out) -> WT9/2(I_in)
  WT1/1(V_out) -> WT2/1(V_in)
  WT3/1(V_out) -> WT4/1(V_in)
  WT4/2(I_out) -> WT3/2(I_in)
  WT4/1(V_out) -> WT5/1(V_in)
  Constant/1 -> WT10/2(I_in)
  WT7/1(V_out) -> WT8/1(V_in)
  WT10/1(V_out) -> Scope/1
